simulink model "vegas01_4tap_1024ch_220_r6c"  (Simulink 7.6) (continued)
== system vegas01_4tap_1024ch_220_r6c == (continued)
  fftshift : xps_library/software register  Tag=xps:sw_reg  SystemSampleTime=-1  io_dir=From Processor  arith_type=Unsigned  bitwidth=32  bin_pt=0  sample_period=1  gw_name=vegas01_4tap_1024ch_220_r6c_fftshift_user_data_out
  gbe0 : xps_library/ten_GbE  Tag=xps:tengbe  SystemSampleTime=-1  port=ROACH:0  mac_lite=off  open_phy=off  show_param=off  pre_emph=3  swing=800  fab_en=off  fab_mac=0  fab_ip=0  fab_udp=0  fab_gate=0
  heap_cntr : xbsIndex_r4/Counter  cnt_type=Free Running  cnt_to=Inf  operation=Up  start_count=0  cnt_by_val=1  arith_type=Unsigned  n_bits=40  bin_pt=0  load_pin=off  rst=on  en=on  explicit_period=on  period=1  dbl_ovrd=off  use_behavioral_HDL=off  implementation=Fabric  block_type=counter
      settings: block_version=11.4
  ip_select : xps_library/software register  Tag=xps:sw_reg  SystemSampleTime=-1  io_dir=From Processor  arith_type=Unsigned  bitwidth=32  bin_pt=0  sample_period=1  gw_name=vegas01_4tap_1024ch_220_r6c_ip_select_user_data_out
      link override r_10gbe_demo_gbe_core_rst_user_data_out: arith_type=Unsigned  n_bits=32  bin_pt=0  period=1
      link override r_10gbe_test_cnt_rst_user_data_out: arith_type=Unsigned  n_bits=32  bin_pt=0  period=1
      link override r_10gbe_test_gbe_core_rst_user_data_out: arith_type=Unsigned  n_bits=32  bin_pt=0  period=1
      link override r_xaui_test_cnt_rst_user_data_out: arith_type=Unsigned  n_bits=32  bin_pt=0  period=1
  length : xps_library/software register  Tag=xps:sw_reg  SystemSampleTime=-1  io_dir=From Processor  arith_type=Unsigned  bitwidth=32  bin_pt=0  sample_period=1  gw_name=vegas01_4tap_1024ch_220_r6c_length_user_data_out
  negedge2 : casper_library_misc/negedge  Tag=Rising Edge Detector  SystemSampleTime=-1
  pfb_fir_real1 : Subsystem  mask(pfb_coeff_gen_dual){pfb_size=11, n_sim_inputs=3, n_taps=4, data_n_bits=8, coeff_n_bits=18, output_bit_width=18, window_fn=hamming, bin_width=1, end_scale_val=1, endDelay=off, cheap_sync=off, autoplace_mode='Attached to ADC0', autoplace_optimize=off, bram_latency_coeff=off, bram_latency_delay=off, register_counter=off, pack_delays=on}
      mask prompts: pfb_size "PFB Size (2^?)"; n_sim_inputs "# Simultaneous Inputs (2^?); Max=6"; n_taps "# Taps"; data_n_bits "Input bit Width"; coeff_n_bits "# Coefficient Bits"; output_bit_width "Output bit width"; window_fn "Window Function"; bin_width "Channel Width"; end_scale_val "Downscale at end (2^?)"; endDelay "Delay at end?"; cheap_sync "Use cheap Sync hardware?"; autoplace_mode "Autoplacement Mode?"; autoplace_optimize "Optimize parameters for auto-placement? (settings below here ignored)"; bram_latency_coeff "Register Coefficient Brams?"; bram_latency_delay "Register Delay Brams?"; register_counter "Register Delay Counters? (0 or 1)"; pack_delays "Pack delays cross-input?"
      mask init:
      | global drawing_parameters;
      | % drawing_parameters.pfb_size=pfb_size;
      | % drawing_parameters.n_sim_inputs=n_sim_inputs;
      | % drawing_parameters.n_taps=n_taps;
      | % drawing_parameters.data_n_bits=data_n_bits;
      | % drawing_parameters.data_bin_pt=data_bin_pt;
      | % drawing_parameters.coeff_n_bits=coeff_n_bits;
      | % drawing_parameters.coeff_bin_pt=coeff_n_bits-1;
      | % drawing_parameters.window_fn=window_fn;
      | % drawing_parameters.mem_type=mem_type;
      | % drawing_parameters.bram_latency=bram_latency;
      | % drawing_parameters.bin_width=bin_width;
      | % drawing_parameters.end_scale_val=end_scale_val;
      | % drawing_parameters.cheap_sync=cheap_sync;
      | config.source=str2func('pfb_improved_draw');
      | config.toplevel=gcb;
      | config.debug=0;
      | config.depend={'pfb_improved_draw.m','pfb_coeff_gen_dual_draw.m'};
      | xBlock(config,{pfb_size,n_sim_inputs,n_taps,data_n_bits,coeff_n_bits, ...
      |                output_bit_width,window_fn,bram_latency_coeff+2,bram_latency_delay+2,bin_width,end_scale_val,endDelay, cheap_sync,register_counter, pack_delays, autoplace_mode, autoplace_optimize});
      mask StyleString: edit,edit,edit,edit,edit,edit,popup(bartlett|barthannwin|blackman|blackmanharris|bohamwin|chebwin|flattopwin|gausswin|hamming|hann|kaiser|nuttallwin|parzenwin|rectwin|tukeywin|triang),edit,edit,checkbox,checkbox,popup('Attached to ADC0'|'Attached to ADC1'|'Attached to both ADC0 and ADC1 (interleaved)'),checkbox,checkbox,checkbox,checkbox,checkbox
  pfb_fir_real3 : Subsystem  mask(pfb_coeff_gen_dual){pfb_size=11, n_sim_inputs=3, n_taps=4, data_n_bits=8, coeff_n_bits=18, output_bit_width=18, window_fn=hamming, bin_width=1, end_scale_val=1, endDelay=off, cheap_sync=off, autoplace_mode='Attached to ADC0', autoplace_optimize=off, bram_latency_coeff=off, bram_latency_delay=off, register_counter=off, pack_delays=on}
      mask prompts: pfb_size "PFB Size (2^?)"; n_sim_inputs "# Simultaneous Inputs (2^?); Max=6"; n_taps "# Taps"; data_n_bits "Input bit Width"; coeff_n_bits "# Coefficient Bits"; output_bit_width "Output bit width"; window_fn "Window Function"; bin_width "Channel Width"; end_scale_val "Downscale at end (2^?)"; endDelay "Delay at end?"; cheap_sync "Use cheap Sync hardware?"; autoplace_mode "Autoplacement Mode?"; autoplace_optimize "Optimize parameters for auto-placement? (settings below here ignored)"; bram_latency_coeff "Register Coefficient Brams?"; bram_latency_delay "Register Delay Brams?"; register_counter "Register Delay Counters? (0 or 1)"; pack_delays "Pack delays cross-input?"
      mask init: same as vegas01_4tap_1024ch_220_r6c/pfb_fir_real1
      mask StyleString: edit,edit,edit,edit,edit,edit,popup(bartlett|barthannwin|blackman|blackmanharris|bohamwin|chebwin|flattopwin|gausswin|hamming|hann|kaiser|nuttallwin|parzenwin|rectwin|tukeywin|triang),edit,edit,checkbox,checkbox,popup('Attached to ADC0'|'Attached to ADC1'|'Attached to both ADC0 and ADC1 (interleaved)'),checkbox,checkbox,checkbox,checkbox,checkbox
  pktsnap : Subsystem  AncestorBlock=casper_library_scopes/snap64  mask(snap64){nsamples=11}
      mask prompts: nsamples "No. of samples(2^?)"
      mask help:
      | The <i>snap</i> block provides a packaged solution to capturing data from
      | the FPGA fabric and making it accessible from the CPU.  The block comes in
      | 2 flavors: <i>snap</i> (which captures to a 32 bit wide Shared BRAM), and
      | <i>snap64</i> (which captures to 2x32 bit wide Shared BRAMs to effect a
      | 64 bit capture).
      mask Help: eval('xlWeb(''http://casper.berkeley.edu/wiki/Snap'')')
  port_select : xps_library/software register  Tag=xps:sw_reg  SystemSampleTime=-1  io_dir=From Processor  arith_type=Unsigned  bitwidth=32  bin_pt=0  sample_period=1  gw_name=vegas01_4tap_1024ch_220_r6c_port_select_user_data_out
      link override r_10gbe_demo_gbe_core_rst_user_data_out: arith_type=Unsigned  n_bits=32  bin_pt=0  period=1
      link override r_10gbe_test_cnt_rst_user_data_out: arith_type=Unsigned  n_bits=32  bin_pt=0  period=1
      link override r_10gbe_test_gbe_core_rst_user_data_out: arith_type=Unsigned  n_bits=32  bin_pt=0  period=1
      link override r_xaui_test_cnt_rst_user_data_out: arith_type=Unsigned  n_bits=32  bin_pt=0  period=1
  posedge : casper_library_misc/posedge  Tag=Rising Edge Detector  SystemSampleTime=-1
  posedge7 : casper_library_misc/posedge  Tag=Rising Edge Detector  SystemSampleTime=-1
  reshaper : Subsystem  mask{inwid=512, inlen=256, outwid=64, outchunk=1024, headlen=10}
      mask prompts: inwid "Input Width (bits)"; inlen "Input Length (clocks)"; outwid "Output Width (bits)"; outchunk "Output Chunk Length (clocks)"; headlen "Header Length"
      mask help:
      | This block takes wide input data and reshapes it to a narrower (or the same)
      | bit width, and breaks it into packets, between which a header can be inserted.
      | Each input data frame must be proceeded by a reset pulse. The input data can be
      | disjoint, with a pulsed valid signal. When the reset signal is pulsed, the previous data
      | frame will be read out. Therefore the number of clocks to read out the header and
      | data must be greater than the number of clocks between two frames (rst signals).
      |
      | The sel signal can be used to drive the select input of a mux which muxes between
      | the data stream (dout) and the indicated number of header words. The header_valid_out
      | signal goes high for each word of the header. dout should be connected to input
      | 0 of the mux. The data_valid_out goes high for each output data value. The st output
      | is for debug access to the internal state machine state.
      mask init:
      | l2inlen = ceil(log2(inlen))
      | l2outchunk = ceil(log2(outchunk))
      | outlen = inlen*(inwid/outwid)
      | nchunk = outlen/outchunk
      | l2headlen = ceil(log2(headlen))
      | l2outlen = ceil(log2(outlen))
  rst : xps_library/software register  Tag=xps:sw_reg  SystemSampleTime=-1  io_dir=From Processor  arith_type=Unsigned  bitwidth=32  bin_pt=0  sample_period=1  gw_name=vegas01_4tap_1024ch_220_r6c_rst_user_data_out
      link override convert: arith_type=Unsigned  n_bits=32  bin_pt=0
      link override r_spec_2048_r102_cnt_rst_user_data_out: arith_type=Unsigned  n_bits=32  bin_pt=0  period=1
      link override r_spec_2048_r102_nosim_cnt_rst_user_data_out: arith_type=Unsigned  n_bits=32  bin_pt=0  period=1
      link override r_spec_2048_r103_cnt_rst_user_data_out: arith_type=Unsigned  n_bits=32  bin_pt=0  period=1
      link override roach_spec_no10gbe_sync_cnt_rst_user_data_out: arith_type=Unsigned  n_bits=32  bin_pt=0  period=1
      link override roach_spec_rst_cnt_user_data_out: arith_type=Unsigned  n_bits=32  bin_pt=0  period=1
      link override roach_spec_rst_sync_cnt_user_data_out: arith_type=Unsigned  n_bits=32  bin_pt=0  period=1
  sg : Subsystem
  speader : Subsystem  AncestorBlock=gbt_library/speader  mask{spead_msw=64, spead_lsw=40, num_items=6, num_payloads=2}
      mask prompts: num_items "Number of items (+5)"; num_payloads "number of payloads"
      mask help:
      | The size of the heap and payload are in Bytes.
      | If there is one packet per heap then payload_offset is 0.
      | All the input must be not longer than 40 bits.
  spect_per : xps_library/software register  Tag=xps:sw_reg  SystemSampleTime=-1  io_dir=From Processor  arith_type=Unsigned  bitwidth=32  bin_pt=0  sample_period=1  gw_name=vegas01_4tap_1024ch_220_r6c_spect_per_user_data_out
  spectrum_cntr : xbsIndex_r4/Counter  cnt_type=Free Running  cnt_to=Inf  operation=Up  start_count=0  cnt_by_val=1  arith_type=Unsigned  n_bits=40  bin_pt=0  load_pin=off  rst=on  en=on  explicit_period=on  period=1  dbl_ovrd=off  use_behavioral_HDL=off  implementation=Fabric  block_type=counter
      settings: block_version=11.4
  ssg_bram : Subsystem  Tag=xps:bram  AncestorBlock=xps_library/Shared BRAM  mask{arith_type=Unsigned, addr_width=11, data_width=32, data_bin_pt=0, init_vals=[12801,121600,3201,3203,57602,3203,3201,121600,12801,12805,121604,3205,3207,57606,3207,3205,121604,12805,0:0], sample_rate=1}
      mask prompts: arith_type "Output Data Type"; addr_width "Address width"; data_width "Data Width"; data_bin_pt "Data Binary Point"; init_vals "Initial 32-bit values (simulation only)"; sample_rate "Sample rate"
      mask init:
      | shared_bram_mask;
      mask StyleString: popup(Unsigned|Signed  (2's comp)),edit,popup(8|16|32|64|128),edit,edit,edit
  stat : Subsystem  AncestorBlock=casper_library_scopes/snap  mask(snap){nsamples=13}
      mask prompts: nsamples "No. of samples(2^?)"
      mask help:
      | The <i>snap</i> block provides a packaged solution to capturing data from
      | the FPGA fabric and making it accessible from the CPU.  The block comes in
      | 2 flavors: <i>snap</i> (which captures to a 32 bit wide Shared BRAM), and
      | <i>snap64</i> (which captures to 2x32 bit wide Shared BRAMs to effect a
      | 64 bit capture).
      mask Help: eval('xlWeb(''http://casper.berkeley.edu/wiki/Snap'')')
  stokes : Subsystem  mask{n_samples=4, addlatency=2}
      mask prompts: n_samples "Number of Simultaneous Inputs"; addlatency "Add Latency"
      mask init:
      | % To make the xBlock smart so it won''t re-generate the
      | % subsystem if neither the arguments nor the scripts are
      | % changes, use as the following:
      | cfg.source = str2func('stokes_init');
      | cfg.toplevel = gcb;
      | cfg.depend = {'stokes_init'};
      | args = {n_samples, addlatency};
      | xBlock(cfg, args);
      mask TabNameString: Basic,Basic
  stokessnap : Subsystem  AncestorBlock=casper_library_scopes/snap64  mask(snap64){nsamples=11}
      mask prompts: nsamples "No. of samples(2^?)"
      mask help:
      | The <i>snap</i> block provides a packaged solution to capturing data from
      | the FPGA fabric and making it accessible from the CPU.  The block comes in
      | 2 flavors: <i>snap</i> (which captures to a 32 bit wide Shared BRAM), and
      | <i>snap64</i> (which captures to 2x32 bit wide Shared BRAMs to effect a
      | 64 bit capture).
      mask Help: eval('xlWeb(''http://casper.berkeley.edu/wiki/Snap'')')
  stokesysnap : Subsystem  AncestorBlock=casper_library_scopes/snap64  mask(snap64){nsamples=11}
      mask prompts: nsamples "No. of samples(2^?)"
      mask help:
      | The <i>snap</i> block provides a packaged solution to capturing data from
      | the FPGA fabric and making it accessible from the CPU.  The block comes in
      | 2 flavors: <i>snap</i> (which captures to a 32 bit wide Shared BRAM), and
      | <i>snap64</i> (which captures to 2x32 bit wide Shared BRAMs to effect a
      | 64 bit capture).
      mask Help: eval('xlWeb(''http://casper.berkeley.edu/wiki/Snap'')')
  time_cntr : xbsIndex_r4/Counter  cnt_type=Free Running  cnt_to=Inf  operation=Up  start_count=0  cnt_by_val=1  arith_type=Unsigned  n_bits=40  bin_pt=0  load_pin=off  rst=on  en=off  explicit_period=on  period=1  dbl_ovrd=off  use_behavioral_HDL=off  implementation=Fabric  block_type=counter
      settings: block_version=11.4
  vacc0 : Subsystem  AncestorBlock=gavrt_library/vacc  mask(vacc){veclen=8, n_inputs=4, max_accum=10, arith_type=1, in_bit_width=32, in_bin_pt=0, out_bit_width=32, out_bin_pt=0, register_din=1, add_latency=2, bram_latency=2, mux_latency=1, use_dsp48=1}
      mask prompts: veclen "Vector Length (2^?)"; n_inputs "Number of Simultaneous Vectors"; max_accum "Maximum # Accumulations (2^?)"; arith_type "Arithmetic Type (0=Unsigned, 1=Signed)"; in_bit_width "Input Bit Width"; in_bin_pt "Input Binary Point"; out_bit_width "Output Bit Width"; out_bin_pt "Output Binary Point"; register_din "Register Input Data  (1 = Enable Register Retiming on input delay)"; add_latency "Add Latency"; bram_latency "BRAM Latency"; mux_latency "Mux Latency"; use_dsp48 "Use DSP48"
      mask help:
      | Sync is expected the clock before the first data to be accumulated.
      | Use the Cram block to feed din for multiple simultaneous inputs.
      | acc_len is the number of vectors to sum.
      | valid is high while a valid accumulation is being clocked out on dout.
      | If only one sync pulse is provided, valid accumulations will repeatedly appear on dout as they become available.
      | Undesired behavior may occur if acc_len is changed without a new sync pulse.
      | Note: The output is an unsigned concatenation of the output streams. Thus, even with only one stream, the output must be recast before using.
      | Register Input Data controls the "Enable Register Retiming" parameter of the delays on 'din'.
      mask init:
      | config.toplevel = gcb;
      | config.source = str2func('vacc_init_xblock');
      | xBlock( config, {...
      |     'veclen', veclen, ...
      |     'n_inputs', n_inputs, ...
      |     'max_accum', max_accum, ...
      |     'arith_type', arith_type, ...
      |     'in_bit_width', in_bit_width, ...
      |     'in_bin_pt', in_bin_pt, ...
      |     'out_bit_width', out_bit_width, ...
      |     'out_bin_pt', out_bin_pt, ...
      |     'register_din', register_din, ...
      |     'add_latency', add_latency, ...
      |     'bram_latency', bram_latency, ...
      |     'mux_latency', mux_latency, ...
      |     'use_dsp48', use_dsp48, ...
      | });
      settings: AttributesFormatString=|
      | vector length=32, inputs=4
      | max accumulations=2^5
  vacc1 : Subsystem  AncestorBlock=gavrt_library/vacc  mask(vacc){veclen=8, n_inputs=4, max_accum=10, arith_type=1, in_bit_width=32, in_bin_pt=0, out_bit_width=32, out_bin_pt=0, register_din=1, add_latency=2, bram_latency=2, mux_latency=1, use_dsp48=1}
      mask prompts: veclen "Vector Length (2^?)"; n_inputs "Number of Simultaneous Vectors"; max_accum "Maximum # Accumulations (2^?)"; arith_type "Arithmetic Type (0=Unsigned, 1=Signed)"; in_bit_width "Input Bit Width"; in_bin_pt "Input Binary Point"; out_bit_width "Output Bit Width"; out_bin_pt "Output Binary Point"; register_din "Register Input Data  (1 = Enable Register Retiming on input delay)"; add_latency "Add Latency"; bram_latency "BRAM Latency"; mux_latency "Mux Latency"; use_dsp48 "Use DSP48"
      mask help:
      | Sync is expected the clock before the first data to be accumulated.
      | Use the Cram block to feed din for multiple simultaneous inputs.
      | acc_len is the number of vectors to sum.
      | valid is high while a valid accumulation is being clocked out on dout.
      | If only one sync pulse is provided, valid accumulations will repeatedly appear on dout as they become available.
      | Undesired behavior may occur if acc_len is changed without a new sync pulse.
      | Note: The output is an unsigned concatenation of the output streams. Thus, even with only one stream, the output must be recast before using.
      | Register Input Data controls the "Enable Register Retiming" parameter of the delays on 'din'.
      mask init: same as vegas01_4tap_1024ch_220_r6c/vacc0
      settings: AttributesFormatString=|
      | vector length=32, inputs=4
      | max accumulations=2^5
  vacc2 : Subsystem  AncestorBlock=gavrt_library/vacc  mask(vacc){veclen=8, n_inputs=4, max_accum=10, arith_type=1, in_bit_width=32, in_bin_pt=0, out_bit_width=32, out_bin_pt=0, register_din=1, add_latency=2, bram_latency=2, mux_latency=1, use_dsp48=1}
      mask prompts: veclen "Vector Length (2^?)"; n_inputs "Number of Simultaneous Vectors"; max_accum "Maximum # Accumulations (2^?)"; arith_type "Arithmetic Type (0=Unsigned, 1=Signed)"; in_bit_width "Input Bit Width"; in_bin_pt "Input Binary Point"; out_bit_width "Output Bit Width"; out_bin_pt "Output Binary Point"; register_din "Register Input Data  (1 = Enable Register Retiming on input delay)"; add_latency "Add Latency"; bram_latency "BRAM Latency"; mux_latency "Mux Latency"; use_dsp48 "Use DSP48"
      mask help:
      | Sync is expected the clock before the first data to be accumulated.
      | Use the Cram block to feed din for multiple simultaneous inputs.
      | acc_len is the number of vectors to sum.
      | valid is high while a valid accumulation is being clocked out on dout.
      | If only one sync pulse is provided, valid accumulations will repeatedly appear on dout as they become available.
      | Undesired behavior may occur if acc_len is changed without a new sync pulse.
      | Note: The output is an unsigned concatenation of the output streams. Thus, even with only one stream, the output must be recast before using.
      | Register Input Data controls the "Enable Register Retiming" parameter of the delays on 'din'.
      mask init: same as vegas01_4tap_1024ch_220_r6c/vacc0
      settings: AttributesFormatString=|
      | vector length=32, inputs=4
      | max accumulations=2^5
  vacc3 : Subsystem  AncestorBlock=gavrt_library/vacc  mask(vacc){veclen=8, n_inputs=4, max_accum=10, arith_type=1, in_bit_width=32, in_bin_pt=0, out_bit_width=32, out_bin_pt=0, register_din=1, add_latency=2, bram_latency=2, mux_latency=1, use_dsp48=1}
      mask prompts: veclen "Vector Length (2^?)"; n_inputs "Number of Simultaneous Vectors"; max_accum "Maximum # Accumulations (2^?)"; arith_type "Arithmetic Type (0=Unsigned, 1=Signed)"; in_bit_width "Input Bit Width"; in_bin_pt "Input Binary Point"; out_bit_width "Output Bit Width"; out_bin_pt "Output Binary Point"; register_din "Register Input Data  (1 = Enable Register Retiming on input delay)"; add_latency "Add Latency"; bram_latency "BRAM Latency"; mux_latency "Mux Latency"; use_dsp48 "Use DSP48"
      mask help:
      | Sync is expected the clock before the first data to be accumulated.
      | Use the Cram block to feed din for multiple simultaneous inputs.
      | acc_len is the number of vectors to sum.
      | valid is high while a valid accumulation is being clocked out on dout.
      | If only one sync pulse is provided, valid accumulations will repeatedly appear on dout as they become available.
      | Undesired behavior may occur if acc_len is changed without a new sync pulse.
      | Note: The output is an unsigned concatenation of the output streams. Thus, even with only one stream, the output must be recast before using.
      | Register Input Data controls the "Enable Register Retiming" parameter of the delays on 'din'.
      mask init: same as vegas01_4tap_1024ch_220_r6c/vacc0
      settings: AttributesFormatString=|
      | vector length=32, inputs=4
      | max accumulations=2^5
  vaccsnap : Subsystem  AncestorBlock=casper_library_scopes/snap64  mask(snap64){nsamples=11}
      mask prompts: nsamples "No. of samples(2^?)"
      mask help:
      | The <i>snap</i> block provides a packaged solution to capturing data from
      | the FPGA fabric and making it accessible from the CPU.  The block comes in
      | 2 flavors: <i>snap</i> (which captures to a 32 bit wide Shared BRAM), and
      | <i>snap64</i> (which captures to 2x32 bit wide Shared BRAMs to effect a
      | 64 bit capture).
      mask Help: eval('xlWeb(''http://casper.berkeley.edu/wiki/Snap'')')
  vaccsynap : Subsystem  AncestorBlock=casper_library_scopes/snap64  mask(snap64){nsamples=11}
      mask prompts: nsamples "No. of samples(2^?)"
      mask help:
      | The <i>snap</i> block provides a packaged solution to capturing data from
      | the FPGA fabric and making it accessible from the CPU.  The block comes in
      | 2 flavors: <i>snap</i> (which captures to a 32 bit wide Shared BRAM), and
      | <i>snap64</i> (which captures to 2x32 bit wide Shared BRAMs to effect a
      | 64 bit capture).
      mask Help: eval('xlWeb(''http://casper.berkeley.edu/wiki/Snap'')')
  wires:
  rst/1 -> Register16/1
  Constant19/1 -> rst/1
  Register16/1 -> Slice2/1
  Slice2/1 -> posedge/1
  posedge/1 -> Delay26/1
  Delay26/1 -> [cnt_rst]
  Register10/1 -> Register40/1
  Register11/1 -> Register41/1
  Register9/1 -> Register39/1
  Register39/1 -> Register38/1
  Register48/1 -> Register73/1
  Register49/1 -> Register74/1
  Register74/1 -> Register27/1
  Register73/1 -> Register26/1
  Register40/1 -> Register17/1
  Register41/1 -> Register23/1
  Constant4/1 -> adc083000x2/4(adc1_data_valid_sim), adc083000x2/3(adc1_sync_sim), adc083000x2/2(adc0_data_valid_sim)
  Constant1/1 -> adc083000x2/1(adc0_sync_sim)
  Slice10/1 -> [adc_sync]
  Register44/1 -> Slice10/1
  adc083000x2/1(adc0_s0) -> [a0]
  adc083000x2/9(adc0_sync) -> Register44/1
  adc083000x2/10(adc0_outofrange) -> Terminator44/1
  adc083000x2/11(adc0_data_valid) -> Terminator45/1
  adc083000x2/12(adc1_s0) -> [b0]
  adc083000x2/20(adc1_sync) -> Terminator43/1
  adc083000x2/21(adc1_outofrange) -> Terminator46/1
  adc083000x2/22(adc1_data_valid) -> Terminator47/1
  Constant20/1 -> adc083000x2/5(sim_adc0_data_in)
  Constant21/1 -> adc083000x2/6(sim_adc1_data_in)
  Concat1/1 -> [default_ip]
  Constant6/1 -> gbe0/7
  gbe0/9 -> Terminator/1
  gbe0/1 -> Terminator17/1
  gbe0/2 -> Terminator16/1
  gbe0/3 -> Terminator15/1
  gbe0/4 -> [txack]
  gbe0/5 -> Terminator8/1
  gbe0/6 -> Terminator6/1
  gbe0/7 -> Terminator5/1
  gbe0/8 -> Terminator3/1
  gbe0/10 -> Terminator11/1
  Constant5/1 -> gbe0/8
  Register38/1 -> Delay1/1
  Delay21/1 -> gbe0/6
  Delay22/1 -> gbe0/5
  Delay24/1 -> gbe0/4
  Delay25/1 -> gbe0/3
  Delay29/1 -> gbe0/2
  Delay30/1 -> gbe0/1
  Constant25/1 -> Mux4/2
  Mux4/1 -> Register22/1
  acc_len/1 -> Mux4/3
  acc_len_sel/1 -> Slice1/1
  Constant26/1 -> acc_len/1
  Register22/1 -> [acc_len]
  Constant3/1 -> acc_len_sel/1
  posedge7/1 -> [anew_acc]
  Slice1/1 -> Mux4/1
  Register17/1 -> [xout0]
  Register23/1 -> [xout1]
  Register26/1 -> [yout0]
  Register27/1 -> [yout1]
  Constant29/1 -> fftshift/1
  fftshift/1 -> Delay53/1
  Delay53/1 -> [fftshift]
  adc083000x2/16(adc1_s4) -> [b4]
  adc083000x2/13(adc1_s1) -> [b1]
  adc083000x2/15(adc1_s3) -> [b3]
  adc083000x2/17(adc1_s5) -> [b5]
  adc083000x2/19(adc1_s7) -> [b7]
  adc083000x2/3(adc0_s2) -> [a2]
  adc083000x2/5(adc0_s4) -> [a4]
  adc083000x2/7(adc0_s6) -> [a6]
  adc083000x2/2(adc0_s1) -> [a1]
  adc083000x2/4(adc0_s3) -> [a3]
  adc083000x2/6(adc0_s5) -> [a5]
  adc083000x2/8(adc0_s7) -> [a7]
  adc083000x2/14(adc1_s2) -> [b2]
  adc083000x2/18(adc1_s6) -> [b6]
  err_clear_sim1/1 -> dest_ip/1
  dest_ip/1 -> [ip_addr]
  dest_port/1 -> Slice7/1
  err_clear_sim3/1 -> dest_port/1
  Slice7/1 -> [port]
  Constant/1 -> Concat1/1
  Constant7/1 -> Concat1/2
  Constant8/1 -> Concat1/3
  Constant9/1 -> Concat1/4
  [default_ip] -> Mux/2
  [ip_addr] -> Mux/3
  err_clear_sim2/1 -> ip_select/1
  ip_select/1 -> Slice8/1
  Slice8/1 -> [ip_select]
  [ip_select] -> Mux/1
  Constant10/1 -> Mux1/2
  [port] -> Mux1/3
  [port_select] -> Mux1/1
  err_clear_sim4/1 -> port_select/1
  port_select/1 -> Slice9/1
  Slice9/1 -> [port_select]
  Mux1/1 -> [dest_port]
  Mux/1 -> [dest_ip]
  Delay1/1 -> [sync_out]
  vacc0/1(valid) -> [valid], posedge7/1
  [cnt_rst] -> Delay30/1
  [data_out] -> speader/1(data_in)
  [data_valid] -> speader/2(valid_in)
  [dest_ip] -> Delay24/1
  [dest_port] -> Delay22/1
  speader/3(eof) -> Delay50/1
  [eof] -> Delay21/1
  Constant18/1 -> speader/3(heap_size)
  Constant33/1 -> speader/8(mode)
  Constant34/1 -> speader/10(payload_offset)
  speader/2(data_valid) -> Delay35/1
  [data_valid_spead] -> Delay25/1
  [data_spead] -> Delay29/1
  heap_cntr/1 -> [heap_cnt]
  Gateway Out10/1 -> Scope8/1
  Gateway Out11/1 -> Scope8/2
  Gateway Out13/1 -> Scope8/3
  [eof] -> Gateway Out11/1
  [data_valid_spead] -> Gateway Out10/1
  [data_spead] -> Gateway Out13/1
  Delay35/1 -> [data_valid_spead]
  [cnt_rst] -> time_cntr/1
  Slice5/1 -> [xxout0]
  Slice6/1 -> [yyout0]
  Slice13/1 -> [xyoutre0]
  Slice18/1 -> [xyoutim0]
  Slice19/1 -> [xxout1]
  Slice20/1 -> [yyout1]
  Slice21/1 -> [xyoutre1]
  Slice22/1 -> [xyoutim1]
  Delay44/1 -> [data_spead]
  [acc_len] -> Convert/1
  Constant24/1 -> speader/4(Packet_length)
  [cnt_rst] -> spectrum_cntr/1
  [eoh] -> spectrum_cntr/2
  [cnt_rst] -> speader/11(Reset)
  Convert/1 -> speader/7(integration size)
  speader/1(data_out) -> Delay44/1
  fft_wideband_real1/1(sync_out) -> Register9/1
  [sync_gen] -> pfb_fir_real1/1(sync)
  [sync_gen] -> pfb_fir_real3/1(sync)
  [a0] -> pfb_fir_real1/2(in0)
  [a1] -> pfb_fir_real1/3(in1)
  [a2] -> pfb_fir_real1/4(in2)
  [a3] -> pfb_fir_real1/5(in3)
  [a4] -> pfb_fir_real1/6(in4)
  [a5] -> pfb_fir_real1/7(in5)
  [a6] -> pfb_fir_real1/8(in6)
  [a7] -> pfb_fir_real1/9(in7)
  [b0] -> pfb_fir_real3/2(in0)
  [b1] -> pfb_fir_real3/3(in1)
  [b2] -> pfb_fir_real3/4(in2)
  [b3] -> pfb_fir_real3/5(in3)
  [b4] -> pfb_fir_real3/6(in4)
  [b5] -> pfb_fir_real3/7(in5)
  [b6] -> pfb_fir_real3/8(in6)
  [b7] -> pfb_fir_real3/9(in7)
  [fftshift] -> Delay5/1
  Delay6/1 -> fft_wideband_real/2(shift)
  Register1/1 -> Register5/1
  Register2/1 -> Register6/1
  Register5/1 -> Register3/1
  Register6/1 -> Register4/1
  Register3/1 -> [xout2]
  Register4/1 -> [xout3]
  fft_wideband_real/1(sync_out) -> Terminator2/1
  Register12/1 -> Register14/1
  Register13/1 -> Register15/1
  Register15/1 -> Register8/1
  Register14/1 -> Register7/1
  Register7/1 -> [yout2]
  Register8/1 -> [yout3]
  [xout0] -> stokes/1(x0)
  [yout0] -> stokes/2(y0)
  [xout1] -> stokes/3(x1)
  [yout1] -> stokes/4(y1)
  [xout2] -> stokes/5(x2)
  [yout2] -> stokes/6(y2)
  [xout3] -> stokes/7(x3)
  [yout3] -> stokes/8(y3)
  Slice29/1 -> [xxout2]
  Slice30/1 -> [yyout2]
  Slice14/1 -> [xyoutre2]
  Slice15/1 -> [xyoutim2]
  Slice25/1 -> [xxout3]
  Slice26/1 -> [yyout3]
  Slice27/1 -> [xyoutre3]
  Slice28/1 -> [xyoutim3]
  stokes/1(x0x0*) -> Slice5/1
  stokes/2(y0y0*) -> Slice6/1
  stokes/3(x0y0*_re) -> Slice13/1
  stokes/4(x0y0*_im) -> Slice18/1
  stokes/5(x1x1*) -> Slice19/1
  stokes/6(y1y1*) -> Slice20/1
  stokes/7(x1y1*_re) -> Slice21/1
  stokes/8(x1y1*_im) -> Slice22/1
  stokes/9(x2x2*) -> Slice29/1
  stokes/10(y2y2*) -> Slice30/1
  stokes/11(x2y2*_re) -> Slice14/1
  stokes/12(x2y2*_im) -> Slice15/1
  stokes/13(x3x3*) -> Slice25/1
  stokes/14(y3y3*) -> Slice26/1
  stokes/15(x3y3*_re) -> Slice27/1
  stokes/16(x3y3*_im) -> Slice28/1
  spectrum_cntr/1 -> [spec_cntr]
  time_cntr/1 -> [time_cntr]
  [time_cntr] -> speader/5(time_stamp)
  [spec_cntr] -> speader/6(spectrum counter)
  [xxout0] -> vacc0/3(din_1_1)
  [xxout1] -> vacc0/4(din_2_1)
  [xxout2] -> vacc0/5(din_3_1)
  [xxout3] -> vacc0/6(din_4_1)
  [yyout0] -> vacc1/3(din_1_1)
  [yyout1] -> vacc1/4(din_2_1)
  [yyout2] -> vacc1/5(din_3_1)
  [yyout3] -> vacc1/6(din_4_1)
  [xyoutre0] -> vacc2/3(din_1_1)
  [xyoutre1] -> vacc2/4(din_2_1)
  [xyoutre2] -> vacc2/5(din_3_1)
  [xyoutre3] -> vacc2/6(din_4_1)
  [xyoutim0] -> vacc3/3(din_1_1)
  [xyoutim1] -> vacc3/4(din_2_1)
  [xyoutim2] -> vacc3/5(din_3_1)
  [xyoutim3] -> vacc3/6(din_4_1)
  cram4/1(Out) -> [din]
  vacc0/2(dout_1_1) -> [xx_vacc0]
  vacc0/3(dout_2_1) -> [xx_vacc1]
  vacc1/2(dout_1_1) -> [yy_vacc0]
  vacc1/3(dout_2_1) -> [yy_vacc1]
  vacc2/2(dout_1_1) -> [xy_vaccre0]
  vacc2/3(dout_2_1) -> [xy_vaccre1]
  vacc3/2(dout_1_1) -> [xy_vaccim0]
  vacc3/3(dout_2_1) -> [xy_vaccim1]
  vacc0/4(dout_3_1) -> [xx_vacc2]
  vacc0/5(dout_4_1) -> [xx_vacc3]
  vacc2/4(dout_3_1) -> [xy_vaccre2]
  vacc2/5(dout_4_1) -> [xy_vaccre3]
  vacc3/4(dout_3_1) -> [xy_vaccim2]
  vacc3/5(dout_4_1) -> [xy_vaccim3]
  [xx_vacc0] -> cram4/1(In1)
  [yy_vacc0] -> cram4/2(In2)
  [xy_vaccre0] -> cram4/3(In3)
  [xy_vaccim0] -> cram4/4(In4)
  [xx_vacc1] -> cram4/5(In5)
  [yy_vacc1] -> cram4/6(In6)
  [xy_vaccre1] -> cram4/7(In7)
  [xy_vaccim1] -> cram4/8(In8)
  [xx_vacc2] -> cram4/9(In9)
  [yy_vacc2] -> cram4/10(In10)
  [xy_vaccre2] -> cram4/11(In11)
  [xy_vaccim2] -> cram4/12(In12)
  [xx_vacc3] -> cram4/13(In13)
  [yy_vacc3] -> cram4/14(In14)
  [xy_vaccre3] -> cram4/15(In15)
  [xy_vaccim3] -> cram4/16(In16)
  Delay50/1 -> [eof]
  [cnt_rst] -> heap_cntr/1
  [eof] -> heap_cntr/2
  speader/4(eoh) -> Delay3/1
  Delay3/1 -> [eoh]
  pfb_fir_real1/2(out0) -> fft_wideband_real1/3(din_0)
  pfb_fir_real1/3(out1) -> fft_wideband_real1/4(din_1)
  pfb_fir_real1/4(out2) -> fft_wideband_real1/5(din_2)
  pfb_fir_real1/5(out3) -> fft_wideband_real1/6(din_3)
  pfb_fir_real1/6(out4) -> fft_wideband_real1/7(din_4)
  pfb_fir_real1/7(out5) -> fft_wideband_real1/8(din_5)
  pfb_fir_real1/8(out6) -> fft_wideband_real1/9(din_6)
  pfb_fir_real1/9(out7) -> fft_wideband_real1/10(din_7)
  pfb_fir_real3/2(out0) -> fft_wideband_real/3(din_0)
  pfb_fir_real3/3(out1) -> fft_wideband_real/4(din_1)
  pfb_fir_real3/4(out2) -> fft_wideband_real/5(din_2)
  pfb_fir_real3/5(out3) -> fft_wideband_real/6(din_3)
  pfb_fir_real3/6(out4) -> fft_wideband_real/7(din_4)
  pfb_fir_real3/7(out5) -> fft_wideband_real/8(din_5)
  pfb_fir_real3/8(out6) -> fft_wideband_real/9(din_6)
  pfb_fir_real3/9(out7) -> fft_wideband_real/10(din_7)
  fft_wideband_real/5(dout3) -> Register13/1
  fft_wideband_real/3(dout1) -> Register49/1
  fft_wideband_real/2(dout0) -> Register48/1
  fft_wideband_real/4(dout2) -> Register12/1
  fft_wideband_real/6(of) -> Terminator30/1
  pfb_fir_real3/1(sync_out) -> fft_wideband_real/1(sync)
  pfb_fir_real1/1(sync_out) -> fft_wideband_real1/1(sync)
  fft_wideband_real1/2(dout0) -> Register10/1
  fft_wideband_real1/3(dout1) -> Register11/1
  fft_wideband_real1/4(dout2) -> Register1/1
  fft_wideband_real1/5(dout3) -> Register2/1
  fft_wideband_real1/6(of) -> Terminator12/1
  [sync_out] -> vacc0/1(sync)
  [acc_len] -> vacc0/2(acc_len)
  [acc_len] -> vacc3/2(acc_len)
  [sync_out] -> vacc1/1(sync)
  [sync_out] -> vacc2/1(sync)
  [sync_out] -> vacc3/1(sync)
  [acc_len] -> vacc2/2(acc_len)
  [acc_len] -> vacc1/2(acc_len)
  vacc1/1(valid) -> Terminator1/1
  vacc2/1(valid) -> Terminator4/1
  vacc3/1(valid) -> Terminator7/1
  vacc1/4(dout_3_1) -> [yy_vacc2]
  vacc1/5(dout_4_1) -> [yy_vacc3]
  reshaper/2(sel) -> Terminator10/1
  reshaper/3(header_valid_out) -> Terminator13/1
  negedge2/1 -> reshaper/1(rst)
  [din] -> Delay13/1
  Delay10/1 -> Delay11/1, negedge2/1
  Delay13/1 -> Delay12/1
  Delay12/1 -> reshaper/3(din)
  Delay11/1 -> reshaper/2(valid)
  reshaper/5(st) -> Terminator14/1
  Delay14/1 -> [data_out]
  Delay15/1 -> [data_valid]
  reshaper/1(dout) -> Delay14/1
  reshaper/4(data_valid_out) -> Delay15/1
  Constant23/1 -> spect_per/1
  Constant11/1 -> length/1
  Constant27/1 -> ssg_bram/2(data_in)
  Constant28/1 -> ssg_bram/3(we)
  length/1 -> CYCLE_LIMIT/2(IN1)
  spect_per/1 -> SP_GEN/1(SP)
  SP_GEN/1(SP_TICK) -> CYCLE_LIMIT/1(IN), DUR_GEN/1(EN)
  DUR_GEN/1(OUT) -> STARTUP/1(IN)
  STARTUP/1(OUT) -> Counter/2
  CYCLE_LIMIT/1(OUT) -> Counter/1
  Counter/1 -> ssg_bram/1(addr)
  ssg_bram/1(data_out) -> SuperSlice/1(In)
  SuperSlice/1(DUR) -> [DUR], DUR_GEN/2(DUR)
  SuperSlice/2(AS/R) -> [ASR]
  SuperSlice/3(S/R1) -> [SR1]
  SuperSlice/4(S/R0) -> [SR0]
  SuperSlice/5(CAL) -> [CAL]
  SuperSlice/6(BLANK) -> [BLANK]
  [BLANK] -> Delay7/1
  [CAL] -> Delay8/1
  [SR0] -> Delay9/1
  [SANITY] -> Delay16/1
  SANITY_SS/1(SANITY) -> [SANITY]
  Constant30/1 -> Register19/1
  Register18/1 -> Inverter/1
  Inverter/1 -> Register19/2  "ppsready"
  Constant31/1 -> Register18/1
  sg/1(sync_out) -> [sync_gen]
  sg/2(pps_out) -> [master_rst]
  arm/1 -> Register20/1
  Constant12/1 -> arm/1
  Register20/1 -> Slice3/1
  Slice3/1 -> [arm]
  [arm] -> Register18/2
  [master_rst] -> Register18/3
  [sync_out] -> Register19/3
  Register19/1 -> [data_enable]
  [valid] -> Logical/1
  [data_enable] -> Logical/2
  Logical/1 -> Delay10/1
  [a0] -> cram/1(In1)
  [a1] -> cram/2(In2)
  [a2] -> cram/3(In3)
  [a3] -> cram/4(In4)
  [a4] -> cram/5(In5)
  [a5] -> cram/6(In6)
  [a6] -> cram/7(In7)
  [a7] -> cram/8(In8)
  cram/1(Out) -> adcsnap/1(din)
  Constant14/1 -> adcsnap/3(we), adcsnap/2(trig)
  cram1/1(Out) -> stokessnap/1(din)
  [xxout0] -> cram1/1(In1)
  [xxout1] -> cram1/2(In2)
  Constant15/1 -> stokessnap/3(we)
  cram3/1(Out) -> vaccsnap/1(din)
  [xx_vacc0] -> cram3/1(In1)
  [xx_vacc1] -> cram3/2(In2)
  [sync_out] -> stokessnap/2(trig)
  [anew_acc] -> vaccsnap/2(trig)
  [valid] -> vaccsnap/3(we)
  [data_spead] -> Delay2/1
  [data_valid_spead] -> pktsnap/3(we)
  [eoh] -> pktsnap/2(trig)
  Delay2/1 -> pktsnap/1(din)
  [data_valid] -> bus_create/2(in2)
  [data_valid_spead] -> bus_create/3(in3)
  [eof] -> bus_create/4(in4)
  [cnt_rst] -> bus_create/5(in5)
  [arm] -> bus_create/6(in6)
  [master_rst] -> bus_create/7(in7)
  [sync_out] -> bus_create/8(in8)
  [data_enable] -> bus_create/9(in9)
  [valid] -> bus_create/10(in10)
  [sync_gen] -> stat/2(trig)
  [data_valid_spead] -> stat/3(we)
  [txack] -> bus_create/1(in1)
  bus_create1/1(bus_out) -> stat/1(din)
  Constant16/1 -> bus_create1/1(in1)
  bus_create/1(bus_out) -> bus_create1/2(in2)
  Constant17/1 -> bus_create2/1(in1)
  bus_create2/1(bus_out) -> Delay4/1
  Delay4/1 -> speader/9(status_bits)
  Delay5/1 -> fft_wideband_real1/2(shift)
  [fftshift] -> Delay6/1
  cram2/1(Out) -> stokesysnap/1(din)
  [yyout0] -> cram2/1(In1)
  [yyout1] -> cram2/2(In2)
  Constant2/1 -> stokesysnap/3(we)
  [sync_out] -> stokesysnap/2(trig)
  cram5/1(Out) -> vaccsynap/1(din)
  [yy_vacc0] -> cram5/1(In1)
  [yy_vacc1] -> cram5/2(In2)
  [anew_acc] -> vaccsynap/2(trig)
  [valid] -> vaccsynap/3(we)
  [adc_sync] -> sg/1(pps)
  [BLANK] -> bus_create2/2(in2)
  [SR0] -> bus_create2/3(in3)
  [CAL] -> bus_create2/4(in4)
  Delay7/1 -> LEDs_GPIOs/1(IN0)
  Delay8/1 -> LEDs_GPIOs/2(IN1)
  Delay9/1 -> LEDs_GPIOs/3(IN2)
  Delay16/1 -> LEDs_GPIOs/4(IN3)
